simulink model "AcasXuClosedLoop_all_comp"  (Simulink 10.0)
settings: Solver=VariableStepAuto  StartTime=0.0  StopTime=20  RelTol=1e-3
libraries: neural x14040, simulink x2
(part 22 of 534)

== system AcasXuClosedLoop_all_comp/networks/Networks_m_5/AcasXu_2_5/Layer 5 ==
  "a{4} " : Inport  PortDimensions=50
      mask Display: e = 0.06;c1 = [120 230 180]/255;c2 = ([120 230 180]+0)/(256+128);c3 = ([120 230 180]+128)/(256+128);patch([0 1 1 0],[0 0 1 1],c1);patch([0 1 1 1-e 1-e 0+e],[0 0 1 1-e 0+e 0+e],c2);patch([0 0 1 1-e 0+e 0+e],[0 1 1 1-e 1-e 0+e],c3);plot([0 1 1 0 0],[0 0 1 1 0]);
  Delays 1 : Subsystem
      mask Display: e = 0.06;c1 = [120 150 230]/255;c2 = ([120 150 230]+0)/383;c3 = ([120 150 230]+128)/383;patch([0 1 1 0],[0 0 1 1],c1);patch([0 1 1 1-e 1-e 0+e],[0 0 1 1-e 0+e 0+e],c2);patch([0 0 1 1-e 0+e 0+e],[0 1 1 1-e 1-e 0+e],c3);plot([0 1 1 0 0],[0 0 1 1 0]);disp('0');
  "LW{5,4}" : Subsystem
      mask Display: e = 0.06;c1 = [120 150 230]/255;c2 = ([120 150 230]+0)/383;c3 = ([120 150 230]+128)/383;patch([0 1 1 0],[0 0 1 1],c1);patch([0 1 1 1-e 1-e 0+e],[0 0 1 1-e 0+e 0+e],c2);patch([0 0 1 1-e 0+e 0+e],[0 1 1 1-e 1-e 0+e],c3);plot([0 1 1 0 0],[0 0 1 1 0]);disp('W');
  "b{5}" : Constant  Value=[-1.113939999999999930224703348358161747455596923828125;-0.93718599999999996352784137343405745923519134521484375;-0.67365399999999997504573912010528147220611572265625;1.8611899999999999000266370785539038479328155517578125;-0.01704920000000000046558312760680564679205417633056640625;-0.438844999999999985096366117431898601353168487548828125;0.33728999999999997871924506398499943315982818603515625;-1.2587200000000000610356210017926059663295745849609375;-0.67630999999999996674659996642731130123138427734375;0.694849000000000049936943469219841063022613525390625;-1.5864000000000000323296944770845584571361541748046875;1.8010200000000000652988774163532070815563201904296875;-3.526949999999999807442918609012849628925323486328125;1.882039999999999935198502498678863048553466796875;0.90225900000000003320366204206948168575763702392578125;-1.4994799999999999240429815472452901303768157958984375;-3.014320000000000110418341137119568884372711181640625;-1.0138499999999999179323140197084285318851470947265625;0.87740399999999996172306282460340298712253570556640625;-1.368559999999999998721023075631819665431976318359375;1.3796999999999999264588268488296307623386383056640625;1.656519999999999992468247000942938029766082763671875;-0.50658400000000003426947614570963196456432342529296875;-0.5171170000000000488427076561492867767810821533203125;-0.8977530000000000232063257499248720705509185791015625;-0.10128299999999999803090844352482235990464687347412109375;-1.82397000000000009123368727159686386585235595703125;-1.11817000000000010828671292983926832675933837890625;2.67096000000000000085265128291212022304534912109375;-2.34762000000000004007461029686965048313140869140625;-1.2765299999999999425170926770078949630260467529296875;-0.0296465999999999986480592184534543775953352451324462890625;0.2625830000000000108428821476991288363933563232421875;0.2130289999999999961399765879832557402551174163818359375;0.296613999999999988776977488669217564165592193603515625;-2.68325000000000013500311979441903531551361083984375;-0.83379899999999995685584508464671671390533447265625;1.411119999999999929940486254054121673107147216796875;0.8879129999999999522941607210668735206127166748046875;1.2620599999999999596411726088263094425201416015625;-0.1891799999999999870592404249691753648221492767333984375;1.47205999999999992411403582082130014896392822265625;-2.65655999999999981042719809920527040958404541015625;1.4213999999999999968025576890795491635799407958984375;-0.51912999999999998035349335623322986066341400146484375;-0.17603299999999999503330627703689970076084136962890625;-0.266799999999999981614706712207407690584659576416015625;-0.79418599999999994754062981883180327713489532470703125;-2.097379999999999800053274157107807695865631103515625;-2.094679999999999875370804147678427398204803466796875]
      mask Display: e = 0.06;c1 = [120 150 230]/255;c2 = ([120 150 230]+0)/383;c3 = ([120 150 230]+128)/383;patch([0 1 1 0],[0 0 1 1],c1);patch([0 1 1 1-e 1-e 0+e],[0 0 1 1-e 0+e 0+e],c2);patch([0 0 1 1-e 0+e 0+e],[0 1 1 1-e 1-e 0+e],c3);plot([0 1 1 0 0],[0 0 1 1 0]);disp('b');
  netsum : Sum  Inputs=2  SaturateOnIntegerOverflow=on  InputSameDT=on  OutDataTypeStr=Inherit: Same as first input
      mask Display: e = 0.06;c1 = [120 150 230]/255;c2 = ([120 150 230]+0)/383;c3 = ([120 150 230]+128)/383;patch([0 1 1 0],[0 0 1 1],c1);patch([0 1 1 1-e 1-e 0+e],[0 0 1 1-e 0+e 0+e],c2);patch([0 0 1 1-e 0+e 0+e],[0 1 1 1-e 1-e 0+e],c3);plot([0 1 1 0 0],[0 0 1 1 0]);disp('+');
  poslin : neural/Transfer Functions/poslin
      settings: SourceProductName=Deep Learning Toolbox  SourceProductBaseCode=NN
  "a{5}" : Outport  InitialOutput=[0;0;0;0;0;0;0;0;0;0;0;0;0;0;0;0;0;0;0;0;0;0;0;0;0;0;0;0;0;0;0;0;0;0;0;0;0;0;0;0;0;0;0;0;0;0;0;0;0;0]
      mask Display: e = 0.06;c1 = [120 230 180]/255;c2 = ([120 230 180]+0)/(256+128);c3 = ([120 230 180]+128)/(256+128);patch([0 1 1 0],[0 0 1 1],c1);patch([0 1 1 1-e 1-e 0+e],[0 0 1 1-e 0+e 0+e],c2);patch([0 0 1 1-e 0+e 0+e],[0 1 1 1-e 1-e 0+e],c3);plot([0 1 1 0 0],[0 0 1 1 0]);
  wires:
  poslin/1 -> "a{5}"/1
  netsum/1 -> poslin/1
  "b{5}"/1 -> netsum/2
  "LW{5,4}"/1("lz{5,4}") -> netsum/1
  Delays 1/1("pd{5,4}") -> "LW{5,4}"/1("ad{5,4}")
  "a{4} "/1 -> Delays 1/1("p{5}")

== system AcasXuClosedLoop_all_comp/networks/Networks_m_5/AcasXu_2_5/Layer 6 ==
  "a{5} " : Inport  PortDimensions=50
      mask Display: e = 0.06;c1 = [120 230 180]/255;c2 = ([120 230 180]+0)/(256+128);c3 = ([120 230 180]+128)/(256+128);patch([0 1 1 0],[0 0 1 1],c1);patch([0 1 1 1-e 1-e 0+e],[0 0 1 1-e 0+e 0+e],c2);patch([0 0 1 1-e 0+e 0+e],[0 1 1 1-e 1-e 0+e],c3);plot([0 1 1 0 0],[0 0 1 1 0]);
  Delays 1 : Subsystem
      mask Display: e = 0.06;c1 = [120 150 230]/255;c2 = ([120 150 230]+0)/383;c3 = ([120 150 230]+128)/383;patch([0 1 1 0],[0 0 1 1],c1);patch([0 1 1 1-e 1-e 0+e],[0 0 1 1-e 0+e 0+e],c2);patch([0 0 1 1-e 0+e 0+e],[0 1 1 1-e 1-e 0+e],c3);plot([0 1 1 0 0],[0 0 1 1 0]);disp('0');
  "LW{6,5}" : Subsystem
      mask Display: e = 0.06;c1 = [120 150 230]/255;c2 = ([120 150 230]+0)/383;c3 = ([120 150 230]+128)/383;patch([0 1 1 0],[0 0 1 1],c1);patch([0 1 1 1-e 1-e 0+e],[0 0 1 1-e 0+e 0+e],c2);patch([0 0 1 1-e 0+e 0+e],[0 1 1 1-e 1-e 0+e],c3);plot([0 1 1 0 0],[0 0 1 1 0]);disp('W');
  "b{6}" : Constant  Value=[-0.261122000000000020758506025231326930224895477294921875;-0.55333699999999996776978150592185556888580322265625;-0.04937539999999999984492404792035813443362712860107421875;-0.433999999999999996891375531049561686813831329345703125;-0.441166000000000002589928271845565177500247955322265625;-0.81616699999999997583444155679899267852306365966796875;-0.159843000000000012850165376221411861479282379150390625;-0.2465819999999999956319385319147841073572635650634765625;0.70091000000000003300471007605665363371372222900390625;0.64531799999999994721378016038215719163417816162109375;0.61738199999999998635047404604847542941570281982421875;0.295640999999999987135623769063386134803295135498046875;0.6789140000000000174651404449832625687122344970703125;0.86423700000000003296207751191104762256145477294921875;0.1776089999999999891056035039582639001309871673583984375;-1.2958499999999999463540234501124359667301177978515625;0.389836000000000015841550293771433643996715545654296875;0.425215999999999982872367354502785019576549530029296875;-0.5226960000000000494679852636181749403476715087890625;0.070630799999999993588062352500855922698974609375;1.5815900000000000513722397954552434384822845458984375;0.03932889999999999997015720509807579219341278076171875;-0.370066999999999979298337393629481084644794464111328125;0.1793019999999999891659996364978724159300327301025390625;-0.04421339999999999992308374885396915487945079803466796875;-0.485640000000000016111556533360271714627742767333984375;-4.8434299999999996799715518136508762836456298828125;0.4636489999999999778168557895696721971035003662109375;-1.4696499999999999008792883614660240709781646728515625;-0.55545900000000003604583298510988242924213409423828125;0.83763299999999996092725496055209077894687652587890625;-0.473893999999999981920240088584250770509243011474609375;0.58603400000000005487521548275253735482692718505859375;0.279193999999999997729815959246479906141757965087890625;0.38427699999999997970689946669153869152069091796875;-0.93696199999999996155253256802097894251346588134765625;0.08268509999999999748876433613986591808497905731201171875;0.02276190000000000157598378791590221226215362548828125;0.1775619999999999976125764078460633754730224609375;-0.0110833000000000007900791132442464004270732402801513671875;-0.179464000000000012402523452692548744380474090576171875;0.698787999999999964728658596868626773357391357421875;-0.4586390000000000188862259165034629404544830322265625;0.0687983000000000066709304746837005950510501861572265625;0.138492000000000003989697461292962543666362762451171875;-0.971338999999999952450480122934095561504364013671875;-0.96719900000000003092992528763716109097003936767578125;0.11212400000000000144240175359300337731838226318359375;-0.076003299999999995861799106933176517486572265625;-0.499443000000000025817570303843240253627300262451171875]
      mask Display: e = 0.06;c1 = [120 150 230]/255;c2 = ([120 150 230]+0)/383;c3 = ([120 150 230]+128)/383;patch([0 1 1 0],[0 0 1 1],c1);patch([0 1 1 1-e 1-e 0+e],[0 0 1 1-e 0+e 0+e],c2);patch([0 0 1 1-e 0+e 0+e],[0 1 1 1-e 1-e 0+e],c3);plot([0 1 1 0 0],[0 0 1 1 0]);disp('b');
  netsum : Sum  Inputs=2  SaturateOnIntegerOverflow=on  InputSameDT=on  OutDataTypeStr=Inherit: Same as first input
      mask Display: e = 0.06;c1 = [120 150 230]/255;c2 = ([120 150 230]+0)/383;c3 = ([120 150 230]+128)/383;patch([0 1 1 0],[0 0 1 1],c1);patch([0 1 1 1-e 1-e 0+e],[0 0 1 1-e 0+e 0+e],c2);patch([0 0 1 1-e 0+e 0+e],[0 1 1 1-e 1-e 0+e],c3);plot([0 1 1 0 0],[0 0 1 1 0]);disp('+');
  poslin : neural/Transfer Functions/poslin
      settings: SourceProductName=Deep Learning Toolbox  SourceProductBaseCode=NN
  "a{6}" : Outport  InitialOutput=[0;0;0;0;0;0;0;0;0;0;0;0;0;0;0;0;0;0;0;0;0;0;0;0;0;0;0;0;0;0;0;0;0;0;0;0;0;0;0;0;0;0;0;0;0;0;0;0;0;0]
      mask Display: e = 0.06;c1 = [120 230 180]/255;c2 = ([120 230 180]+0)/(256+128);c3 = ([120 230 180]+128)/(256+128);patch([0 1 1 0],[0 0 1 1],c1);patch([0 1 1 1-e 1-e 0+e],[0 0 1 1-e 0+e 0+e],c2);patch([0 0 1 1-e 0+e 0+e],[0 1 1 1-e 1-e 0+e],c3);plot([0 1 1 0 0],[0 0 1 1 0]);
  wires:
  poslin/1 -> "a{6}"/1
  netsum/1 -> poslin/1
  "b{6}"/1 -> netsum/2
  "LW{6,5}"/1("lz{6,5}") -> netsum/1
  Delays 1/1("pd{6,5}") -> "LW{6,5}"/1("ad{6,5}")
  "a{5} "/1 -> Delays 1/1("p{6}")

== system AcasXuClosedLoop_all_comp/networks/Networks_m_5/AcasXu_2_5/Layer 7 ==
  "a{6} " : Inport  PortDimensions=50
      mask Display: e = 0.06;c1 = [120 230 180]/255;c2 = ([120 230 180]+0)/(256+128);c3 = ([120 230 180]+128)/(256+128);patch([0 1 1 0],[0 0 1 1],c1);patch([0 1 1 1-e 1-e 0+e],[0 0 1 1-e 0+e 0+e],c2);patch([0 0 1 1-e 0+e 0+e],[0 1 1 1-e 1-e 0+e],c3);plot([0 1 1 0 0],[0 0 1 1 0]);
  Delays 1 : Subsystem
      mask Display: e = 0.06;c1 = [120 150 230]/255;c2 = ([120 150 230]+0)/383;c3 = ([120 150 230]+128)/383;patch([0 1 1 0],[0 0 1 1],c1);patch([0 1 1 1-e 1-e 0+e],[0 0 1 1-e 0+e 0+e],c2);patch([0 0 1 1-e 0+e 0+e],[0 1 1 1-e 1-e 0+e],c3);plot([0 1 1 0 0],[0 0 1 1 0]);disp('0');
  "LW{7,6}" : Subsystem
      mask Display: e = 0.06;c1 = [120 150 230]/255;c2 = ([120 150 230]+0)/383;c3 = ([120 150 230]+128)/383;patch([0 1 1 0],[0 0 1 1],c1);patch([0 1 1 1-e 1-e 0+e],[0 0 1 1-e 0+e 0+e],c2);patch([0 0 1 1-e 0+e 0+e],[0 1 1 1-e 1-e 0+e],c3);plot([0 1 1 0 0],[0 0 1 1 0]);disp('W');
  "b{7}" : Constant  Value=[-0.020287800000000001665600990463644848205149173736572265625;-0.0189644999999999987194687633973444462753832340240478515625;0.0186505999999999998173461079886692459695041179656982421875;-0.01901819999999999899475966458339826203882694244384765625;0.0184603999999999983383514035040207090787589550018310546875]
      mask Display: e = 0.06;c1 = [120 150 230]/255;c2 = ([120 150 230]+0)/383;c3 = ([120 150 230]+128)/383;patch([0 1 1 0],[0 0 1 1],c1);patch([0 1 1 1-e 1-e 0+e],[0 0 1 1-e 0+e 0+e],c2);patch([0 0 1 1-e 0+e 0+e],[0 1 1 1-e 1-e 0+e],c3);plot([0 1 1 0 0],[0 0 1 1 0]);disp('b');
  netsum : Sum  Inputs=2  SaturateOnIntegerOverflow=on  InputSameDT=on  OutDataTypeStr=Inherit: Same as first input
      mask Display: e = 0.06;c1 = [120 150 230]/255;c2 = ([120 150 230]+0)/383;c3 = ([120 150 230]+128)/383;patch([0 1 1 0],[0 0 1 1],c1);patch([0 1 1 1-e 1-e 0+e],[0 0 1 1-e 0+e 0+e],c2);patch([0 0 1 1-e 0+e 0+e],[0 1 1 1-e 1-e 0+e],c3);plot([0 1 1 0 0],[0 0 1 1 0]);disp('+');
  purelin : neural/Transfer Functions/purelin
      settings: SourceProductName=Deep Learning Toolbox
  "a{7}" : Outport  InitialOutput=[0;0;0;0;0]
      mask Display: e = 0.06;c1 = [120 230 180]/255;c2 = ([120 230 180]+0)/(256+128);c3 = ([120 230 180]+128)/(256+128);patch([0 1 1 0],[0 0 1 1],c1);patch([0 1 1 1-e 1-e 0+e],[0 0 1 1-e 0+e 0+e],c2);patch([0 0 1 1-e 0+e 0+e],[0 1 1 1-e 1-e 0+e],c3);plot([0 1 1 0 0],[0 0 1 1 0]);
  wires:
  purelin/1 -> "a{7}"/1
  netsum/1 -> purelin/1
  "b{7}"/1 -> netsum/2
  "LW{7,6}"/1("lz{7,6}") -> netsum/1
  Delays 1/1("pd{7,6}") -> "LW{7,6}"/1("ad{7,6}")
  "a{6} "/1 -> Delays 1/1("p{7}")

== system AcasXuClosedLoop_all_comp/networks/Networks_m_5/AcasXu_2_5/Process Input 1 ==
  x : Inport  PortDimensions=5
      mask Display: e = 0.06;c1 = [120 230 180]/255;c2 = ([120 230 180]+0)/(256+128);c3 = ([120 230 180]+128)/(256+128);patch([0 1 1 0],[0 0 1 1],c1);patch([0 1 1 1-e 1-e 0+e],[0 0 1 1-e 0+e 0+e],c2);patch([0 0 1 1-e 0+e 0+e],[0 1 1 1-e 1-e 0+e],c3);plot([0 1 1 0 0],[0 0 1 1 0]);
  p : Outport  PortDimensions=5
      mask Display: e = 0.06;c1 = [120 230 180]/255;c2 = ([120 230 180]+0)/(256+128);c3 = ([120 230 180]+128)/(256+128);patch([0 1 1 0],[0 0 1 1],c1);patch([0 1 1 1-e 1-e 0+e],[0 0 1 1-e 0+e 0+e],c2);patch([0 0 1 1-e 0+e 0+e],[0 1 1 1-e 1-e 0+e],c3);plot([0 1 1 0 0],[0 0 1 1 0]);
  wires:
  x/1 -> p/1

== system AcasXuClosedLoop_all_comp/networks/Networks_m_5/AcasXu_2_5/Process Output 1 ==
  a : Inport  PortDimensions=5
      mask Display: e = 0.06;c1 = [120 230 180]/255;c2 = ([120 230 180]+0)/(256+128);c3 = ([120 230 180]+128)/(256+128);patch([0 1 1 0],[0 0 1 1],c1);patch([0 1 1 1-e 1-e 0+e],[0 0 1 1-e 0+e 0+e],c2);patch([0 0 1 1-e 0+e 0+e],[0 1 1 1-e 1-e 0+e],c3);plot([0 1 1 0 0],[0 0 1 1 0]);
  y : Outport  PortDimensions=5
      mask Display: e = 0.06;c1 = [120 230 180]/255;c2 = ([120 230 180]+0)/(256+128);c3 = ([120 230 180]+128)/(256+128);patch([0 1 1 0],[0 0 1 1],c1);patch([0 1 1 1-e 1-e 0+e],[0 0 1 1-e 0+e 0+e],c2);patch([0 0 1 1-e 0+e 0+e],[0 1 1 1-e 1-e 0+e],c3);plot([0 1 1 0 0],[0 0 1 1 0]);
  wires:
  a/1 -> y/1

== system AcasXuClosedLoop_all_comp/networks/Networks_m_5/AcasXu_3_5/Layer 1 ==
  "p{1}" : Inport  PortDimensions=5
      mask Display: e = 0.06;c1 = [120 230 180]/255;c2 = ([120 230 180]+0)/(256+128);c3 = ([120 230 180]+128)/(256+128);patch([0 1 1 0],[0 0 1 1],c1);patch([0 1 1 1-e 1-e 0+e],[0 0 1 1-e 0+e 0+e],c2);patch([0 0 1 1-e 0+e 0+e],[0 1 1 1-e 1-e 0+e],c3);plot([0 1 1 0 0],[0 0 1 1 0]);
  Delays 1 : Subsystem
      mask Display: e = 0.06;c1 = [120 150 230]/255;c2 = ([120 150 230]+0)/383;c3 = ([120 150 230]+128)/383;patch([0 1 1 0],[0 0 1 1],c1);patch([0 1 1 1-e 1-e 0+e],[0 0 1 1-e 0+e 0+e],c2);patch([0 0 1 1-e 0+e 0+e],[0 1 1 1-e 1-e 0+e],c3);plot([0 1 1 0 0],[0 0 1 1 0]);disp('0');
  "IW{1,1}" : Subsystem
      mask Display: e = 0.06;c1 = [120 150 230]/255;c2 = ([120 150 230]+0)/383;c3 = ([120 150 230]+128)/383;patch([0 1 1 0],[0 0 1 1],c1);patch([0 1 1 1-e 1-e 0+e],[0 0 1 1-e 0+e 0+e],c2);patch([0 0 1 1-e 0+e 0+e],[0 1 1 1-e 1-e 0+e],c3);plot([0 1 1 0 0],[0 0 1 1 0]);disp('W');
  "b{1}" : Constant  Value=[-0.0158440999999999998504307541224989108741283416748046875;-0.442506000000000010441425501994672231376171112060546875;0.06955350000000000421085388779829372651875019073486328125;0.01255460000000000082842621651479930733330547809600830078125;-0.32413999999999998369304421430570073425769805908203125;-0.187265999999999988023802188763511367142200469970703125;-0.281405999999999989480414797071716748178005218505859375;-0.52566900000000005288569582262425683438777923583984375;-0.2210809999999999997388755446081631816923618316650390625;0.20538000000000000699884594723698683083057403564453125;-0.332755999999999996230570786792668513953685760498046875;-0.1848160000000000080522255530013353563845157623291015625;0.134469000000000005190514684727531857788562774658203125;-0.00103063000000000004434397293806568995933048427104949951171875;0.1990010000000000112141407271337811835110187530517578125;0.11770899999999999419042495674148085527122020721435546875;-0.282907999999999992812860227786586619913578033447265625;0.12504999999999999449329379785922355949878692626953125;-0.7286129999999999551363316641072742640972137451171875;0.26820699999999997320543343448662199079990386962890625;-0.442798999999999998156141600702540017664432525634765625;0.09730060000000000097752916872195783071219921112060546875;-0.06887759999999999716013832085081958211958408355712890625;0.089545399999999997220356817706488072872161865234375;-0.27821200000000001484323775002849288284778594970703125;0.07353400000000000213962181305760168470442295074462890625;-0.336774999999999991029397961028735153377056121826171875;-0.01361109999999999924102933590575048583559691905975341796875;0.037762700000000003208722176850642426870763301849365234375;0.292630000000000001225686219186172820627689361572265625;0.1157870000000000010320633236915455199778079986572265625;0.3744899999999999895550217843265272676944732666015625;-0.01042389999999999983248955004455638118088245391845703125;0.3699040000000000105728759081102907657623291015625;0.68385200000000001541167193863657303154468536376953125;-0.11929299999999999626343338832157314755022525787353515625;-0.045373900000000001730970922153574065305292606353759765625;0.094742300000000001514166569904773496091365814208984375;0.214926000000000005929479129918036051094532012939453125;-0.1308749999999999913402604079237789846956729888916015625;-0.152426000000000005929479129918036051094532012939453125;-0.0180756000000000006944222974425429129041731357574462890625;0.0910270999999999996799004975400748662650585174560546875;0.037993800000000001182431930146776721812784671783447265625;-0.28277099999999999457855892615043558180332183837890625;-0.0446290000000000020019541580040822736918926239013671875;0.283007999999999981799447823505033738911151885986328125;-0.48900799999999999823074858795735053718090057373046875;0.1318539999999999989821475310236564837396144866943359375;0.0013348100000000000762978569213146329275332391262054443359375]
      mask Display: e = 0.06;c1 = [120 150 230]/255;c2 = ([120 150 230]+0)/383;c3 = ([120 150 230]+128)/383;patch([0 1 1 0],[0 0 1 1],c1);patch([0 1 1 1-e 1-e 0+e],[0 0 1 1-e 0+e 0+e],c2);patch([0 0 1 1-e 0+e 0+e],[0 1 1 1-e 1-e 0+e],c3);plot([0 1 1 0 0],[0 0 1 1 0]);disp('b');
  netsum : Sum  Inputs=2  SaturateOnIntegerOverflow=on  InputSameDT=on  OutDataTypeStr=Inherit: Same as first input
      mask Display: e = 0.06;c1 = [120 150 230]/255;c2 = ([120 150 230]+0)/383;c3 = ([120 150 230]+128)/383;patch([0 1 1 0],[0 0 1 1],c1);patch([0 1 1 1-e 1-e 0+e],[0 0 1 1-e 0+e 0+e],c2);patch([0 0 1 1-e 0+e 0+e],[0 1 1 1-e 1-e 0+e],c3);plot([0 1 1 0 0],[0 0 1 1 0]);disp('+');
  poslin : neural/Transfer Functions/poslin
      settings: SourceProductName=Deep Learning Toolbox  SourceProductBaseCode=NN
  "a{1}" : Outport  InitialOutput=[0;0;0;0;0;0;0;0;0;0;0;0;0;0;0;0;0;0;0;0;0;0;0;0;0;0;0;0;0;0;0;0;0;0;0;0;0;0;0;0;0;0;0;0;0;0;0;0;0;0]
      mask Display: e = 0.06;c1 = [120 230 180]/255;c2 = ([120 230 180]+0)/(256+128);c3 = ([120 230 180]+128)/(256+128);patch([0 1 1 0],[0 0 1 1],c1);patch([0 1 1 1-e 1-e 0+e],[0 0 1 1-e 0+e 0+e],c2);patch([0 0 1 1-e 0+e 0+e],[0 1 1 1-e 1-e 0+e],c3);plot([0 1 1 0 0],[0 0 1 1 0]);
  wires:
  poslin/1 -> "a{1}"/1
  netsum/1 -> poslin/1
  "b{1}"/1 -> netsum/2
  "IW{1,1}"/1("iz{1,1}") -> netsum/1
  Delays 1/1("pd{1,1}") -> "IW{1,1}"/1("pd{1,1}")
  "p{1}"/1 -> Delays 1/1("p{1}")

== system AcasXuClosedLoop_all_comp/networks/Networks_m_5/AcasXu_3_5/Layer 2 ==
  "a{1} " : Inport  PortDimensions=50
      mask Display: e = 0.06;c1 = [120 230 180]/255;c2 = ([120 230 180]+0)/(256+128);c3 = ([120 230 180]+128)/(256+128);patch([0 1 1 0],[0 0 1 1],c1);patch([0 1 1 1-e 1-e 0+e],[0 0 1 1-e 0+e 0+e],c2);patch([0 0 1 1-e 0+e 0+e],[0 1 1 1-e 1-e 0+e],c3);plot([0 1 1 0 0],[0 0 1 1 0]);
  Delays 1 : Subsystem
      mask Display: e = 0.06;c1 = [120 150 230]/255;c2 = ([120 150 230]+0)/383;c3 = ([120 150 230]+128)/383;patch([0 1 1 0],[0 0 1 1],c1);patch([0 1 1 1-e 1-e 0+e],[0 0 1 1-e 0+e 0+e],c2);patch([0 0 1 1-e 0+e 0+e],[0 1 1 1-e 1-e 0+e],c3);plot([0 1 1 0 0],[0 0 1 1 0]);disp('0');
  "LW{2,1}" : Subsystem
      mask Display: e = 0.06;c1 = [120 150 230]/255;c2 = ([120 150 230]+0)/383;c3 = ([120 150 230]+128)/383;patch([0 1 1 0],[0 0 1 1],c1);patch([0 1 1 1-e 1-e 0+e],[0 0 1 1-e 0+e 0+e],c2);patch([0 0 1 1-e 0+e 0+e],[0 1 1 1-e 1-e 0+e],c3);plot([0 1 1 0 0],[0 0 1 1 0]);disp('W');
  "b{2}" : Constant  Value=[-0.4764829999999999898818714427761733531951904296875;1.176509999999999944719775157864205539226531982421875;-0.770413000000000014466650100075639784336090087890625;-0.018943200000000000315925063887334545142948627471923828125;-0.756812000000000040245140553452074527740478515625;0.83248599999999994825117255459190346300601959228515625;-0.8530320000000000124629195852321572601795196533203125;0.049777700000000001112621106358346878550946712493896484375;-0.46842600000000000903810359886847436428070068359375;-0.870778000000000051983306548208929598331451416015625;-1.211440000000000072333250500378198921680450439453125;-0.781908000000000047435833039344288408756256103515625;-0.005312889999999999800228689394998582429252564907073974609375;-0.398832000000000019834800468743196688592433929443359375;-0.362281999999999992922994351829402148723602294921875;-0.258964000000000027501556587594677694141864776611328125;0.57258500000000001062261389961349777877330780029296875;-0.004214899999999999723188093270209719776175916194915771484375;-0.017670100000000001083666489876122795976698398590087890625;0.2388659999999999949960027834094944410026073455810546875;-0.1529979999999999951132423348099109716713428497314453125;0.334548000000000012033041230097296647727489471435546875;0.0157225999999999997702726517445626086555421352386474609375;-0.0089733399999999997886934721691432059742510318756103515625;-1.1960100000000000175504055732744745910167694091796875;0.565514999999999989910293152206577360630035400390625;0.384514999999999995683452880257391370832920074462890625;-1.286589999999999900381908446433953940868377685546875;-1.1185899999999999732125388618442229926586151123046875;0.5638039999999999718482968091848306357860565185546875;-0.5968750000000000444089209850062616169452667236328125;0.0734347999999999945242024068647879175841808319091796875;-0.6251670000000000282369683191063813865184783935546875;-0.1766000000000000069722005946459830738604068756103515625;-0.15633900000000000574118530494160950183868408203125;-0.01309330000000000042426062663025732035748660564422607421875;-0.021993300000000000349320572468059253878891468048095703125;0.92117700000000002358291339987772516906261444091796875;-0.423219000000000011851852832478471100330352783203125;-0.59943800000000002636824092405731789767742156982421875;-0.5829100000000000392219590139575302600860595703125;-0.01278080000000000014670487047396818525157868862152099609375;-0.06572619999999999851336696110593038611114025115966796875;-0.99215299999999995161914512209477834403514862060546875;-0.5792450000000000098765440270653925836086273193359375;-0.0086539300000000006496403415212625986896455287933349609375;0.1974579999999999946336259881718433462083339691162109375;-0.333199999999999996180832795289461500942707061767578125;-0.01305890000000000002178257574314557132311165332794189453125;-1.4259500000000000508038056068471632897853851318359375]
      mask Display: e = 0.06;c1 = [120 150 230]/255;c2 = ([120 150 230]+0)/383;c3 = ([120 150 230]+128)/383;patch([0 1 1 0],[0 0 1 1],c1);patch([0 1 1 1-e 1-e 0+e],[0 0 1 1-e 0+e 0+e],c2);patch([0 0 1 1-e 0+e 0+e],[0 1 1 1-e 1-e 0+e],c3);plot([0 1 1 0 0],[0 0 1 1 0]);disp('b');
  netsum : Sum  Inputs=2  SaturateOnIntegerOverflow=on  InputSameDT=on  OutDataTypeStr=Inherit: Same as first input
      mask Display: e = 0.06;c1 = [120 150 230]/255;c2 = ([120 150 230]+0)/383;c3 = ([120 150 230]+128)/383;patch([0 1 1 0],[0 0 1 1],c1);patch([0 1 1 1-e 1-e 0+e],[0 0 1 1-e 0+e 0+e],c2);patch([0 0 1 1-e 0+e 0+e],[0 1 1 1-e 1-e 0+e],c3);plot([0 1 1 0 0],[0 0 1 1 0]);disp('+');
  poslin : neural/Transfer Functions/poslin
      settings: SourceProductName=Deep Learning Toolbox  SourceProductBaseCode=NN
  "a{2}" : Outport  InitialOutput=[0;0;0;0;0;0;0;0;0;0;0;0;0;0;0;0;0;0;0;0;0;0;0;0;0;0;0;0;0;0;0;0;0;0;0;0;0;0;0;0;0;0;0;0;0;0;0;0;0;0]
      mask Display: e = 0.06;c1 = [120 230 180]/255;c2 = ([120 230 180]+0)/(256+128);c3 = ([120 230 180]+128)/(256+128);patch([0 1 1 0],[0 0 1 1],c1);patch([0 1 1 1-e 1-e 0+e],[0 0 1 1-e 0+e 0+e],c2);patch([0 0 1 1-e 0+e 0+e],[0 1 1 1-e 1-e 0+e],c3);plot([0 1 1 0 0],[0 0 1 1 0]);
  wires:
  poslin/1 -> "a{2}"/1
  netsum/1 -> poslin/1
  "b{2}"/1 -> netsum/2
  "LW{2,1}"/1("lz{2,1}") -> netsum/1
  Delays 1/1("pd{2,1}") -> "LW{2,1}"/1("ad{2,1}")
  "a{1} "/1 -> Delays 1/1("p{2}")

== system AcasXuClosedLoop_all_comp/networks/Networks_m_5/AcasXu_3_5/Layer 3 ==
  "a{2} " : Inport  PortDimensions=50
      mask Display: e = 0.06;c1 = [120 230 180]/255;c2 = ([120 230 180]+0)/(256+128);c3 = ([120 230 180]+128)/(256+128);patch([0 1 1 0],[0 0 1 1],c1);patch([0 1 1 1-e 1-e 0+e],[0 0 1 1-e 0+e 0+e],c2);patch([0 0 1 1-e 0+e 0+e],[0 1 1 1-e 1-e 0+e],c3);plot([0 1 1 0 0],[0 0 1 1 0]);
  Delays 1 : Subsystem
      mask Display: e = 0.06;c1 = [120 150 230]/255;c2 = ([120 150 230]+0)/383;c3 = ([120 150 230]+128)/383;patch([0 1 1 0],[0 0 1 1],c1);patch([0 1 1 1-e 1-e 0+e],[0 0 1 1-e 0+e 0+e],c2);patch([0 0 1 1-e 0+e 0+e],[0 1 1 1-e 1-e 0+e],c3);plot([0 1 1 0 0],[0 0 1 1 0]);disp('0');
  "LW{3,2}" : Subsystem
      mask Display: e = 0.06;c1 = [120 150 230]/255;c2 = ([120 150 230]+0)/383;c3 = ([120 150 230]+128)/383;patch([0 1 1 0],[0 0 1 1],c1);patch([0 1 1 1-e 1-e 0+e],[0 0 1 1-e 0+e 0+e],c2);patch([0 0 1 1-e 0+e 0+e],[0 1 1 1-e 1-e 0+e],c3);plot([0 1 1 0 0],[0 0 1 1 0]);disp('W');
  "b{3}" : Constant  Value=[0.10803300000000000402611277650066767819225788116455078125;-0.2349860000000000004316547119742608629167079925537109375;-0.639329000000000036152414395473897457122802734375;-0.005685059999999999592878996423905846313573420047760009765625;0.10558800000000000129940502802128321491181850433349609375;-0.323882999999999976470377305304282344877719879150390625;1.251069999999999904360947766690514981746673583984375;-1.575860000000000038511416278197430074214935302734375;0.11748699999999999421529395249308436177670955657958984375;-1.78258999999999989682919476763345301151275634765625;0.049596300000000002883293603872516541741788387298583984375;1.766059999999999963193886287626810371875762939453125;-0.171536999999999995036858990715700201690196990966796875;-0.179976999999999998092192754484131000936031341552734375;-0.024822299999999998532107525761603028513491153717041015625;-1.5661300000000000220978790821391157805919647216796875;0.6232480000000000242010855799890123307704925537109375;0.297680000000000000159872115546022541821002960205078125;-2.1084399999999998698285708087496459484100341796875;-0.0183017000000000006065814517342005274258553981781005859375;-0.323564000000000018264501022713375277817249298095703125;-2.538510000000000044195758164278231561183929443359375;-1.2244099999999999983657517077517695724964141845703125;-0.498294999999999987938537060472299344837665557861328125;-0.1761070000000000135287336888723075389862060546875;-0.510348000000000023845814212108962237834930419921875;-0.40757799999999999585043042316101491451263427734375;-0.04525490000000000068158811927787610329687595367431640625;0.060115700000000001079758504829442244954407215118408203125;-1.416469999999999895834434937569312751293182373046875;-0.03441070000000000239648301203487790189683437347412109375;-1.0618600000000000260769184023956768214702606201171875;0.275598000000000009634959496906958520412445068359375;-1.568820000000000103312913779518567025661468505859375;-0.18839200000000000390087961932295002043247222900390625;-2.7531400000000001426769813406281173229217529296875;1.84515999999999991132426657713949680328369140625;-0.362485000000000001652011860642232932150363922119140625;-0.08782040000000000679047928997533745132386684417724609375;-0.11232799999999999729372035517371841706335544586181640625;1.0437000000000000721200876796501688659191131591796875;-0.0029449900000000001944189254032835378893651068210601806640625;-1.681059999999999998721023075631819665431976318359375;-0.65200499999999994571453498792834579944610595703125;1.0705899999999999305799747162382118403911590576171875;-0.319740999999999997438493437584838829934597015380859375;-0.1786750000000000004884981308350688777863979339599609375;-1.280839999999999978541609380044974386692047119140625;-0.63481100000000001415401129634119570255279541015625;-1.520399999999999973709918776876293122768402099609375]
      mask Display: e = 0.06;c1 = [120 150 230]/255;c2 = ([120 150 230]+0)/383;c3 = ([120 150 230]+128)/383;patch([0 1 1 0],[0 0 1 1],c1);patch([0 1 1 1-e 1-e 0+e],[0 0 1 1-e 0+e 0+e],c2);patch([0 0 1 1-e 0+e 0+e],[0 1 1 1-e 1-e 0+e],c3);plot([0 1 1 0 0],[0 0 1 1 0]);disp('b');
  netsum : Sum  Inputs=2  SaturateOnIntegerOverflow=on  InputSameDT=on  OutDataTypeStr=Inherit: Same as first input
      mask Display: e = 0.06;c1 = [120 150 230]/255;c2 = ([120 150 230]+0)/383;c3 = ([120 150 230]+128)/383;patch([0 1 1 0],[0 0 1 1],c1);patch([0 1 1 1-e 1-e 0+e],[0 0 1 1-e 0+e 0+e],c2);patch([0 0 1 1-e 0+e 0+e],[0 1 1 1-e 1-e 0+e],c3);plot([0 1 1 0 0],[0 0 1 1 0]);disp('+');
  poslin : neural/Transfer Functions/poslin
      settings: SourceProductName=Deep Learning Toolbox  SourceProductBaseCode=NN
  "a{3}" : Outport  InitialOutput=[0;0;0;0;0;0;0;0;0;0;0;0;0;0;0;0;0;0;0;0;0;0;0;0;0;0;0;0;0;0;0;0;0;0;0;0;0;0;0;0;0;0;0;0;0;0;0;0;0;0]
      mask Display: e = 0.06;c1 = [120 230 180]/255;c2 = ([120 230 180]+0)/(256+128);c3 = ([120 230 180]+128)/(256+128);patch([0 1 1 0],[0 0 1 1],c1);patch([0 1 1 1-e 1-e 0+e],[0 0 1 1-e 0+e 0+e],c2);patch([0 0 1 1-e 0+e 0+e],[0 1 1 1-e 1-e 0+e],c3);plot([0 1 1 0 0],[0 0 1 1 0]);
  wires:
  poslin/1 -> "a{3}"/1
  netsum/1 -> poslin/1
  "b{3}"/1 -> netsum/2
  "LW{3,2}"/1("lz{3,2}") -> netsum/1
  Delays 1/1("pd{3,2}") -> "LW{3,2}"/1("ad{3,2}")
  "a{2} "/1 -> Delays 1/1("p{3}")

== system AcasXuClosedLoop_all_comp/networks/Networks_m_5/AcasXu_3_5/Layer 4 ==
  "a{3} " : Inport  PortDimensions=50
      mask Display: e = 0.06;c1 = [120 230 180]/255;c2 = ([120 230 180]+0)/(256+128);c3 = ([120 230 180]+128)/(256+128);patch([0 1 1 0],[0 0 1 1],c1);patch([0 1 1 1-e 1-e 0+e],[0 0 1 1-e 0+e 0+e],c2);patch([0 0 1 1-e 0+e 0+e],[0 1 1 1-e 1-e 0+e],c3);plot([0 1 1 0 0],[0 0 1 1 0]);
  Delays 1 : Subsystem
      mask Display: e = 0.06;c1 = [120 150 230]/255;c2 = ([120 150 230]+0)/383;c3 = ([120 150 230]+128)/383;patch([0 1 1 0],[0 0 1 1],c1);patch([0 1 1 1-e 1-e 0+e],[0 0 1 1-e 0+e 0+e],c2);patch([0 0 1 1-e 0+e 0+e],[0 1 1 1-e 1-e 0+e],c3);plot([0 1 1 0 0],[0 0 1 1 0]);disp('0');
  "LW{4,3}" : Subsystem
      mask Display: e = 0.06;c1 = [120 150 230]/255;c2 = ([120 150 230]+0)/383;c3 = ([120 150 230]+128)/383;patch([0 1 1 0],[0 0 1 1],c1);patch([0 1 1 1-e 1-e 0+e],[0 0 1 1-e 0+e 0+e],c2);patch([0 0 1 1-e 0+e 0+e],[0 1 1 1-e 1-e 0+e],c3);plot([0 1 1 0 0],[0 0 1 1 0]);disp('W');
  "b{4}" : Constant  Value=[-0.036469300000000003103561851958147599361836910247802734375;-2.01231999999999988659737937268801033496856689453125;-1.8681300000000000682831569065456278622150421142578125;0.83909900000000003927880243281833827495574951171875;-0.544950000000000045474735088646411895751953125;0.2541189999999999837854147699545137584209442138671875;-0.9948069999999999968309793985099531710147857666015625;-0.2263179999999999914006565404633875004947185516357421875;0.093010800000000004583711188388406299054622650146484375;-1.6856999999999999761968183520366437733173370361328125;0.343113999999999974566122773467213846743106842041015625;2.160530000000000061533000916824676096439361572265625;-0.8875159999999999715925014243111945688724517822265625;-1.6759999999999999342747969421907328069210052490234375;-0.8696260000000000101039177025086246430873870849609375;-1.4873300000000000409272615797817707061767578125;1.7875900000000000122923893286497332155704498291015625;0.275874000000000008103739901343942619860172271728515625;-0.6970739999999999714219711677287705242633819580078125;-0.262917000000000011805667554654064588248729705810546875;-0.50441199999999997150013086866238154470920562744140625;0.7083040000000000446789272245950996875762939453125;0.92541700000000004511235829340876080095767974853515625;-0.1302750000000000019095836023552692495286464691162109375;-0.0165786999999999982990939173532751738093793392181396484375;0.430375000000000007549516567451064474880695343017578125;1.750220000000000108997255665599368512630462646484375;1.330529999999999990478727340814657509326934814453125;0.5249960000000000182041048901737667620182037353515625;0.10622499999999999997779553950749686919152736663818359375;1.28960000000000007958078640513122081756591796875;-1.0654600000000000736832816983223892748355865478515625;-0.6910650000000000403588273911736905574798583984375;-0.6329380000000000006110667527536861598491668701171875;0.12304299999999999959410246219704276882112026214599609375;-1.2885899999999999021582652858342044055461883544921875;-0.0300354000000000002923439268442962202243506908416748046875;-0.11562799999999999467359401705834898166358470916748046875;-1.48730999999999990990318110561929643154144287109375;-0.12274300000000000487876405941278790123760700225830078125;-0.06206129999999999979909404146383167244493961334228515625;0.86320399999999997131538975736475549638271331787109375;-1.10724000000000000198951966012828052043914794921875;-0.1317899999999999904876091250116587616503238677978515625;-2.072649999999999881339363128063268959522247314453125;-0.399527000000000021007195982747361995279788970947265625;0.82006199999999995764454752134042792022228240966796875;-1.20655000000000001136868377216160297393798828125;-0.1266380000000000005666578317686798982322216033935546875;1.0789899999999998936317524567130021750926971435546875]
      mask Display: e = 0.06;c1 = [120 150 230]/255;c2 = ([120 150 230]+0)/383;c3 = ([120 150 230]+128)/383;patch([0 1 1 0],[0 0 1 1],c1);patch([0 1 1 1-e 1-e 0+e],[0 0 1 1-e 0+e 0+e],c2);patch([0 0 1 1-e 0+e 0+e],[0 1 1 1-e 1-e 0+e],c3);plot([0 1 1 0 0],[0 0 1 1 0]);disp('b');
  netsum : Sum  Inputs=2  SaturateOnIntegerOverflow=on  InputSameDT=on  OutDataTypeStr=Inherit: Same as first input
      mask Display: e = 0.06;c1 = [120 150 230]/255;c2 = ([120 150 230]+0)/383;c3 = ([120 150 230]+128)/383;patch([0 1 1 0],[0 0 1 1],c1);patch([0 1 1 1-e 1-e 0+e],[0 0 1 1-e 0+e 0+e],c2);patch([0 0 1 1-e 0+e 0+e],[0 1 1 1-e 1-e 0+e],c3);plot([0 1 1 0 0],[0 0 1 1 0]);disp('+');
  poslin : neural/Transfer Functions/poslin
      settings: SourceProductName=Deep Learning Toolbox  SourceProductBaseCode=NN
  "a{4}" : Outport  InitialOutput=[0;0;0;0;0;0;0;0;0;0;0;0;0;0;0;0;0;0;0;0;0;0;0;0;0;0;0;0;0;0;0;0;0;0;0;0;0;0;0;0;0;0;0;0;0;0;0;0;0;0]
      mask Display: e = 0.06;c1 = [120 230 180]/255;c2 = ([120 230 180]+0)/(256+128);c3 = ([120 230 180]+128)/(256+128);patch([0 1 1 0],[0 0 1 1],c1);patch([0 1 1 1-e 1-e 0+e],[0 0 1 1-e 0+e 0+e],c2);patch([0 0 1 1-e 0+e 0+e],[0 1 1 1-e 1-e 0+e],c3);plot([0 1 1 0 0],[0 0 1 1 0]);
  wires:
  poslin/1 -> "a{4}"/1
  netsum/1 -> poslin/1
  "b{4}"/1 -> netsum/2
  "LW{4,3}"/1("lz{4,3}") -> netsum/1
  Delays 1/1("pd{4,3}") -> "LW{4,3}"/1("ad{4,3}")
  "a{3} "/1 -> Delays 1/1("p{4}")

== system AcasXuClosedLoop_all_comp/networks/Networks_m_5/AcasXu_3_5/Layer 5 ==
  "a{4} " : Inport  PortDimensions=50
      mask Display: e = 0.06;c1 = [120 230 180]/255;c2 = ([120 230 180]+0)/(256+128);c3 = ([120 230 180]+128)/(256+128);patch([0 1 1 0],[0 0 1 1],c1);patch([0 1 1 1-e 1-e 0+e],[0 0 1 1-e 0+e 0+e],c2);patch([0 0 1 1-e 0+e 0+e],[0 1 1 1-e 1-e 0+e],c3);plot([0 1 1 0 0],[0 0 1 1 0]);
  Delays 1 : Subsystem
      mask Display: e = 0.06;c1 = [120 150 230]/255;c2 = ([120 150 230]+0)/383;c3 = ([120 150 230]+128)/383;patch([0 1 1 0],[0 0 1 1],c1);patch([0 1 1 1-e 1-e 0+e],[0 0 1 1-e 0+e 0+e],c2);patch([0 0 1 1-e 0+e 0+e],[0 1 1 1-e 1-e 0+e],c3);plot([0 1 1 0 0],[0 0 1 1 0]);disp('0');
  "LW{5,4}" : Subsystem
      mask Display: e = 0.06;c1 = [120 150 230]/255;c2 = ([120 150 230]+0)/383;c3 = ([120 150 230]+128)/383;patch([0 1 1 0],[0 0 1 1],c1);patch([0 1 1 1-e 1-e 0+e],[0 0 1 1-e 0+e 0+e],c2);patch([0 0 1 1-e 0+e 0+e],[0 1 1 1-e 1-e 0+e],c3);plot([0 1 1 0 0],[0 0 1 1 0]);disp('W');
  "b{5}" : Constant  Value=[1.35179999999999989057641869294457137584686279296875;-1.4148400000000000975575176198617555201053619384765625;0.422458999999999973429254396251053549349308013916015625;-0.2712689999999999823643292984343133866786956787109375;-0.59447799999999995090860238633467815816402435302734375;-1.3994500000000000827782287160516716539859771728515625;-1.6830400000000000915179043659009039402008056640625;2.68742999999999998550492819049395620822906494140625;0.43552299999999999347011225836467929184436798095703125;-1.6252999999999999669597627871553413569927215576171875;-1.5141400000000000414246414948138408362865447998046875;0.251269000000000020111912135689635761082172393798828125;-0.260417999999999982829734790357179008424282073974609375;0.2129330000000000111537445945941726677119731903076171875;0.3357959999999999833875108379288576543331146240234375;0.857326000000000032486013878951780498027801513671875;1.93803999999999998493649400188587605953216552734375;-0.315282000000000006689759857181343249976634979248046875;-0.6832030000000000047322146201622672379016876220703125;0.6761719999999999952677853798377327620983123779296875;1.113869999999999915729631538852117955684661865234375;-3.2187999999999998834709913353435695171356201171875;0.4285010000000000207620587389101274311542510986328125;0.71382299999999998529887079712352715432643890380859375;-0.1590169999999999916884263484462280757725238800048828125;1.397110000000000074038553066202439367771148681640625;1.358109999999999928377292235381901264190673828125;-1.975470000000000059259264162392355501651763916015625;-0.345362000000000002319922032256727106869220733642578125;2.499470000000000080575546235195361077785491943359375;2.297489999999999810142981004901230335235595703125;0.313798999999999994603427921902039088308811187744140625;0.8608759999999999745767809145036153495311737060546875;0.514529000000000014125589586910791695117950439453125;0.5111980000000000412541112382314167916774749755859375;0.75193600000000004879296966464607976377010345458984375;-0.143739000000000005652367462971596978604793548583984375;0.14651300000000000434141611549421213567256927490234375;-1.388149999999999995026200849679298698902130126953125;0.298667999999999989046983728258055634796619415283203125;-0.1808079999999999964988006695421063341200351715087890625;-0.01647389999999999965485386610453133471310138702392578125;-0.6454750000000000209610107049229554831981658935546875;-1.887939999999999951540985421161167323589324951171875;-0.761414999999999952962070892681367695331573486328125;0.84787500000000004529709940470638684928417205810546875;0.50677000000000005375255796025157906115055084228515625;-0.59009900000000004016698085251846350729465484619140625;-1.50724999999999997868371792719699442386627197265625;1.377520000000000077733375292154960334300994873046875]
      mask Display: e = 0.06;c1 = [120 150 230]/255;c2 = ([120 150 230]+0)/383;c3 = ([120 150 230]+128)/383;patch([0 1 1 0],[0 0 1 1],c1);patch([0 1 1 1-e 1-e 0+e],[0 0 1 1-e 0+e 0+e],c2);patch([0 0 1 1-e 0+e 0+e],[0 1 1 1-e 1-e 0+e],c3);plot([0 1 1 0 0],[0 0 1 1 0]);disp('b');
  netsum : Sum  Inputs=2  SaturateOnIntegerOverflow=on  InputSameDT=on  OutDataTypeStr=Inherit: Same as first input
      mask Display: e = 0.06;c1 = [120 150 230]/255;c2 = ([120 150 230]+0)/383;c3 = ([120 150 230]+128)/383;patch([0 1 1 0],[0 0 1 1],c1);patch([0 1 1 1-e 1-e 0+e],[0 0 1 1-e 0+e 0+e],c2);patch([0 0 1 1-e 0+e 0+e],[0 1 1 1-e 1-e 0+e],c3);plot([0 1 1 0 0],[0 0 1 1 0]);disp('+');
  poslin : neural/Transfer Functions/poslin
      settings: SourceProductName=Deep Learning Toolbox  SourceProductBaseCode=NN
  "a{5}" : Outport  InitialOutput=[0;0;0;0;0;0;0;0;0;0;0;0;0;0;0;0;0;0;0;0;0;0;0;0;0;0;0;0;0;0;0;0;0;0;0;0;0;0;0;0;0;0;0;0;0;0;0;0;0;0]
      mask Display: e = 0.06;c1 = [120 230 180]/255;c2 = ([120 230 180]+0)/(256+128);c3 = ([120 230 180]+128)/(256+128);patch([0 1 1 0],[0 0 1 1],c1);patch([0 1 1 1-e 1-e 0+e],[0 0 1 1-e 0+e 0+e],c2);patch([0 0 1 1-e 0+e 0+e],[0 1 1 1-e 1-e 0+e],c3);plot([0 1 1 0 0],[0 0 1 1 0]);
  wires:
  poslin/1 -> "a{5}"/1
  netsum/1 -> poslin/1
  "b{5}"/1 -> netsum/2
  "LW{5,4}"/1("lz{5,4}") -> netsum/1
  Delays 1/1("pd{5,4}") -> "LW{5,4}"/1("ad{5,4}")
  "a{4} "/1 -> Delays 1/1("p{5}")

== system AcasXuClosedLoop_all_comp/networks/Networks_m_5/AcasXu_3_5/Layer 6 ==
  "a{5} " : Inport  PortDimensions=50
      mask Display: e = 0.06;c1 = [120 230 180]/255;c2 = ([120 230 180]+0)/(256+128);c3 = ([120 230 180]+128)/(256+128);patch([0 1 1 0],[0 0 1 1],c1);patch([0 1 1 1-e 1-e 0+e],[0 0 1 1-e 0+e 0+e],c2);patch([0 0 1 1-e 0+e 0+e],[0 1 1 1-e 1-e 0+e],c3);plot([0 1 1 0 0],[0 0 1 1 0]);
  Delays 1 : Subsystem
      mask Display: e = 0.06;c1 = [120 150 230]/255;c2 = ([120 150 230]+0)/383;c3 = ([120 150 230]+128)/383;patch([0 1 1 0],[0 0 1 1],c1);patch([0 1 1 1-e 1-e 0+e],[0 0 1 1-e 0+e 0+e],c2);patch([0 0 1 1-e 0+e 0+e],[0 1 1 1-e 1-e 0+e],c3);plot([0 1 1 0 0],[0 0 1 1 0]);disp('0');
  "LW{6,5}" : Subsystem
      mask Display: e = 0.06;c1 = [120 150 230]/255;c2 = ([120 150 230]+0)/383;c3 = ([120 150 230]+128)/383;patch([0 1 1 0],[0 0 1 1],c1);patch([0 1 1 1-e 1-e 0+e],[0 0 1 1-e 0+e 0+e],c2);patch([0 0 1 1-e 0+e 0+e],[0 1 1 1-e 1-e 0+e],c3);plot([0 1 1 0 0],[0 0 1 1 0]);disp('W');
  "b{6}" : Constant  Value=[-2.51630999999999982463805281440727412700653076171875;-0.75749999999999995115018691649311222136020660400390625;-4.80323000000000011056044968427158892154693603515625;0.133898999999999990251353665371425449848175048828125;-0.60562700000000002642508434291812591254711151123046875;-0.80418299999999998117772292971494607627391815185546875;-0.95463299999999995382182760295108892023563385009765625;-0.50059200000000003694111683216760866343975067138671875;-1.818449999999999899813474257825873792171478271484375;-1.519330000000000069348971010185778141021728515625;-1.221999999999999975131004248396493494510650634765625;-0.10571099999999999941024952931911684572696685791015625;-0.11281499999999999861444166526780463755130767822265625;-0.184892000000000000792255150372511707246303558349609375;-0.7979760000000000186304305316298268735408782958984375;-0.08334080000000000654214460382718243636190891265869140625;-0.06550809999999999966302510756577248685061931610107421875;-0.25310100000000002040678737103007733821868896484375;-0.32285799999999997833555198667454533278942108154296875;-0.1656209999999999904485292745448532514274120330810546875;0.021539200000000001400390914341187453828752040863037109375;-0.59008400000000005292122295941226184368133544921875;-1.3424400000000000776623210185789503157138824462890625;0.12439799999999999469135758545235148631036281585693359375;0.246304999999999996163069226895458996295928955078125;0.25622699999999998254907040973193943500518798828125;-0.934312999999999949096718410146422684192657470703125;-0.461334999999999995079491554861306212842464447021484375;-0.7360860000000000180619963430217467248439788818359375;-0.4330760000000000164988023243495263159275054931640625;-2.02728000000000019298340703244321048259735107421875;0.052405100000000003068745257905902690254151821136474609375;0.045652400000000002699973578046410693787038326263427734375;-5.27383999999999986130205797962844371795654296875;-3.2795100000000001472244548494927585124969482421875;0.090838900000000000201083594220108352601528167724609375;-0.337550999999999989942267575315781868994235992431640625;0.2137819999999999998063771045053726993501186370849609375;-3.375080000000000080007112046587280929088592529296875;-0.258249000000000006327383061943692155182361602783203125;0.10914600000000000690203449948967318050563335418701171875;-0.44719100000000000516564568897592835128307342529296875;-0.12049300000000000288036261508750612847506999969482421875;-0.12132700000000000428723723189250449649989604949951171875;0.14462099999999999955235807647113688290119171142578125;-1.0978399999999999270272610374377109110355377197265625;-6.388600000000000278532752417959272861480712890625;-0.03508229999999999682014362178961164318025112152099609375;-1.0352799999999999780442294650129042565822601318359375;-0.670517999999999947391415844322182238101959228515625]
      mask Display: e = 0.06;c1 = [120 150 230]/255;c2 = ([120 150 230]+0)/383;c3 = ([120 150 230]+128)/383;patch([0 1 1 0],[0 0 1 1],c1);patch([0 1 1 1-e 1-e 0+e],[0 0 1 1-e 0+e 0+e],c2);patch([0 0 1 1-e 0+e 0+e],[0 1 1 1-e 1-e 0+e],c3);plot([0 1 1 0 0],[0 0 1 1 0]);disp('b');
  netsum : Sum  Inputs=2  SaturateOnIntegerOverflow=on  InputSameDT=on  OutDataTypeStr=Inherit: Same as first input
      mask Display: e = 0.06;c1 = [120 150 230]/255;c2 = ([120 150 230]+0)/383;c3 = ([120 150 230]+128)/383;patch([0 1 1 0],[0 0 1 1],c1);patch([0 1 1 1-e 1-e 0+e],[0 0 1 1-e 0+e 0+e],c2);patch([0 0 1 1-e 0+e 0+e],[0 1 1 1-e 1-e 0+e],c3);plot([0 1 1 0 0],[0 0 1 1 0]);disp('+');
  poslin : neural/Transfer Functions/poslin
      settings: SourceProductName=Deep Learning Toolbox  SourceProductBaseCode=NN
  "a{6}" : Outport  InitialOutput=[0;0;0;0;0;0;0;0;0;0;0;0;0;0;0;0;0;0;0;0;0;0;0;0;0;0;0;0;0;0;0;0;0;0;0;0;0;0;0;0;0;0;0;0;0;0;0;0;0;0]
      mask Display: e = 0.06;c1 = [120 230 180]/255;c2 = ([120 230 180]+0)/(256+128);c3 = ([120 230 180]+128)/(256+128);patch([0 1 1 0],[0 0 1 1],c1);patch([0 1 1 1-e 1-e 0+e],[0 0 1 1-e 0+e 0+e],c2);patch([0 0 1 1-e 0+e 0+e],[0 1 1 1-e 1-e 0+e],c3);plot([0 1 1 0 0],[0 0 1 1 0]);
  wires:
  poslin/1 -> "a{6}"/1
  netsum/1 -> poslin/1
  "b{6}"/1 -> netsum/2
  "LW{6,5}"/1("lz{6,5}") -> netsum/1
  Delays 1/1("pd{6,5}") -> "LW{6,5}"/1("ad{6,5}")
  "a{5} "/1 -> Delays 1/1("p{6}")

== system AcasXuClosedLoop_all_comp/networks/Networks_m_5/AcasXu_3_5/Layer 7 ==
  "a{6} " : Inport  PortDimensions=50
      mask Display: e = 0.06;c1 = [120 230 180]/255;c2 = ([120 230 180]+0)/(256+128);c3 = ([120 230 180]+128)/(256+128);patch([0 1 1 0],[0 0 1 1],c1);patch([0 1 1 1-e 1-e 0+e],[0 0 1 1-e 0+e 0+e],c2);patch([0 0 1 1-e 0+e 0+e],[0 1 1 1-e 1-e 0+e],c3);plot([0 1 1 0 0],[0 0 1 1 0]);
  Delays 1 : Subsystem
      mask Display: e = 0.06;c1 = [120 150 230]/255;c2 = ([120 150 230]+0)/383;c3 = ([120 150 230]+128)/383;patch([0 1 1 0],[0 0 1 1],c1);patch([0 1 1 1-e 1-e 0+e],[0 0 1 1-e 0+e 0+e],c2);patch([0 0 1 1-e 0+e 0+e],[0 1 1 1-e 1-e 0+e],c3);plot([0 1 1 0 0],[0 0 1 1 0]);disp('0');
  "LW{7,6}" : Subsystem
      mask Display: e = 0.06;c1 = [120 150 230]/255;c2 = ([120 150 230]+0)/383;c3 = ([120 150 230]+128)/383;patch([0 1 1 0],[0 0 1 1],c1);patch([0 1 1 1-e 1-e 0+e],[0 0 1 1-e 0+e 0+e],c2);patch([0 0 1 1-e 0+e 0+e],[0 1 1 1-e 1-e 0+e],c3);plot([0 1 1 0 0],[0 0 1 1 0]);disp('W');
  "b{7}" : Constant  Value=[-0.0208699000000000002563727008464411483146250247955322265625;0.0183713999999999995360600024696395848877727985382080078125;-0.02015329999999999899262803637611796148121356964111328125;0.018250599999999998768185349717896315269172191619873046875;-0.0182586999999999992361221501369072939269244670867919921875]
      mask Display: e = 0.06;c1 = [120 150 230]/255;c2 = ([120 150 230]+0)/383;c3 = ([120 150 230]+128)/383;patch([0 1 1 0],[0 0 1 1],c1);patch([0 1 1 1-e 1-e 0+e],[0 0 1 1-e 0+e 0+e],c2);patch([0 0 1 1-e 0+e 0+e],[0 1 1 1-e 1-e 0+e],c3);plot([0 1 1 0 0],[0 0 1 1 0]);disp('b');
  netsum : Sum  Inputs=2  SaturateOnIntegerOverflow=on  InputSameDT=on  OutDataTypeStr=Inherit: Same as first input
      mask Display: e = 0.06;c1 = [120 150 230]/255;c2 = ([120 150 230]+0)/383;c3 = ([120 150 230]+128)/383;patch([0 1 1 0],[0 0 1 1],c1);patch([0 1 1 1-e 1-e 0+e],[0 0 1 1-e 0+e 0+e],c2);patch([0 0 1 1-e 0+e 0+e],[0 1 1 1-e 1-e 0+e],c3);plot([0 1 1 0 0],[0 0 1 1 0]);disp('+');
  purelin : neural/Transfer Functions/purelin
      settings: SourceProductName=Deep Learning Toolbox
  "a{7}" : Outport  InitialOutput=[0;0;0;0;0]
      mask Display: e = 0.06;c1 = [120 230 180]/255;c2 = ([120 230 180]+0)/(256+128);c3 = ([120 230 180]+128)/(256+128);patch([0 1 1 0],[0 0 1 1],c1);patch([0 1 1 1-e 1-e 0+e],[0 0 1 1-e 0+e 0+e],c2);patch([0 0 1 1-e 0+e 0+e],[0 1 1 1-e 1-e 0+e],c3);plot([0 1 1 0 0],[0 0 1 1 0]);
  wires:
  purelin/1 -> "a{7}"/1
  netsum/1 -> purelin/1
  "b{7}"/1 -> netsum/2
  "LW{7,6}"/1("lz{7,6}") -> netsum/1
  Delays 1/1("pd{7,6}") -> "LW{7,6}"/1("ad{7,6}")
  "a{6} "/1 -> Delays 1/1("p{7}")

== system AcasXuClosedLoop_all_comp/networks/Networks_m_5/AcasXu_3_5/Process Input 1 ==
  x : Inport  PortDimensions=5
      mask Display: e = 0.06;c1 = [120 230 180]/255;c2 = ([120 230 180]+0)/(256+128);c3 = ([120 230 180]+128)/(256+128);patch([0 1 1 0],[0 0 1 1],c1);patch([0 1 1 1-e 1-e 0+e],[0 0 1 1-e 0+e 0+e],c2);patch([0 0 1 1-e 0+e 0+e],[0 1 1 1-e 1-e 0+e],c3);plot([0 1 1 0 0],[0 0 1 1 0]);
  p : Outport  PortDimensions=5
      mask Display: e = 0.06;c1 = [120 230 180]/255;c2 = ([120 230 180]+0)/(256+128);c3 = ([120 230 180]+128)/(256+128);patch([0 1 1 0],[0 0 1 1],c1);patch([0 1 1 1-e 1-e 0+e],[0 0 1 1-e 0+e 0+e],c2);patch([0 0 1 1-e 0+e 0+e],[0 1 1 1-e 1-e 0+e],c3);plot([0 1 1 0 0],[0 0 1 1 0]);
  wires:
  x/1 -> p/1
